simulink model "benchmark"  (Simulink 7.9)
settings: Solver=ode45  StartTime=0.0  StopTime=1000  RelTol=1e-3
undefined symbols (not defined in the file or in its folder): sigbuilder_block
libraries: libturbines x10, simulink x1
(part 2 of 3)

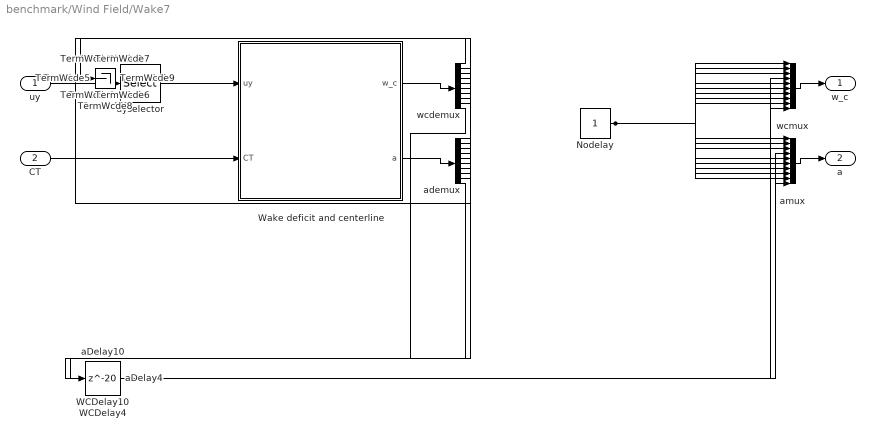
== system benchmark/Wind Field/Wake7 ==
  uy : Inport
  CT : Inport  Port=2
  Nodelay : Constant
  TermAde{1..3,5..9} (8 alike) : Terminator
  TermWcde{1..3,5..9} (8 alike) : Terminator
  WCDelay10 : Delay  DelayLength=20  InitialCondition=1500  InputProcessing=Inherited  SampleTime=1
  WCDelay4 : Delay  DelayLength=20  InitialCondition=1500  InputProcessing=Inherited  SampleTime=1
  Wake deficit and centerline : MATLAB Function  TreatAsAtomicUnit=on
  aDelay10 : Delay  DelayLength=20  InitialCondition=1  InputProcessing=Inherited  SampleTime=1
  aDelay4 : Delay  DelayLength=20  InitialCondition=1  InputProcessing=Inherited  SampleTime=1
  ademux : Demux  Outputs=10
  amux : Mux  Inputs=10
  uyselector : Selector  NumberOfDimensions=2  InputPortWidth=3  IndexOptions=Select all,Index vector (dialog)  Indices=[1 3],p.wt.num  OutputSizes=1,1
  wcdemux : Demux  Outputs=10
  wcmux : Mux  Inputs=10
  w_c : Outport
  a : Outport  Port=2
  wires:
  amux/1 -> a/1
  wcmux/1 -> w_c/1
  Wake deficit and centerline/2(a) -> ademux/1
  Wake deficit and centerline/1(w_c) -> wcdemux/1
  uyselector/1 -> Wake deficit and centerline/1(uy)
  uy/1 -> uyselector/1
  CT/1 -> Wake deficit and centerline/2(CT)
  ademux/1 -> TermAde1/1
  wcdemux/1 -> TermWcde1/1
  Nodelay/1 -> amux/1, wcmux/1, amux/2, wcmux/2, amux/3, wcmux/3, amux/5, wcmux/5, amux/6, wcmux/6, amux/7, wcmux/7, amux/8, wcmux/8, amux/9, wcmux/9
  ademux/2 -> TermAde2/1
  wcdemux/2 -> TermWcde2/1
  ademux/3 -> TermAde3/1
  wcdemux/3 -> TermWcde3/1
  ademux/4 -> aDelay4/1
  aDelay4/1 -> amux/4
  wcdemux/4 -> WCDelay4/1
  WCDelay4/1 -> wcmux/4
  ademux/5 -> TermAde5/1
  wcdemux/5 -> TermWcde5/1
  ademux/6 -> TermAde6/1
  wcdemux/6 -> TermWcde6/1
  ademux/7 -> TermAde7/1
  wcdemux/7 -> TermWcde7/1
  ademux/8 -> TermAde8/1
  wcdemux/8 -> TermWcde8/1
  ademux/9 -> TermAde9/1
  wcdemux/9 -> TermWcde9/1
  ademux/10 -> aDelay10/1
  aDelay10/1 -> amux/10
  wcdemux/10 -> WCDelay10/1
  WCDelay10/1 -> wcmux/10

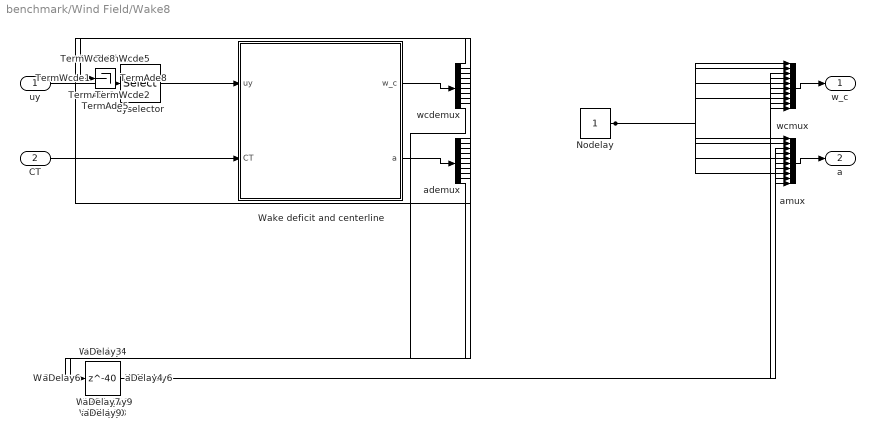
== system benchmark/Wind Field/Wake8 ==
  uy : Inport
  CT : Inport  Port=2
  Nodelay : Constant
  TermAde{1..2,5,8} (4 alike) : Terminator
  TermWcde{1..2,5,8} (4 alike) : Terminator
  WCDelay10 : Delay  DelayLength=80  InitialCondition=2000  InputProcessing=Inherited  SampleTime=1
  WCDelay3 : Delay  DelayLength=40  InitialCondition=2000  InputProcessing=Inherited  SampleTime=1
  WCDelay4 : Delay  DelayLength=80  InitialCondition=2000  InputProcessing=Inherited  SampleTime=1
  WCDelay6 : Delay  DelayLength=20  InitialCondition=2000  InputProcessing=Inherited  SampleTime=1
  WCDelay7 : Delay  DelayLength=60  InitialCondition=2000  InputProcessing=Inherited  SampleTime=1
  WCDelay9 : Delay  DelayLength=40  InitialCondition=2000  InputProcessing=Inherited  SampleTime=1
  Wake deficit and centerline : MATLAB Function  TreatAsAtomicUnit=on
  aDelay10 : Delay  DelayLength=80  InitialCondition=1  InputProcessing=Inherited  SampleTime=1
  aDelay3 : Delay  DelayLength=40  InitialCondition=1  InputProcessing=Inherited  SampleTime=1
  aDelay4 : Delay  DelayLength=80  InitialCondition=1  InputProcessing=Inherited  SampleTime=1
  aDelay6 : Delay  DelayLength=20  InitialCondition=1  InputProcessing=Inherited  SampleTime=1
  aDelay7 : Delay  DelayLength=60  InitialCondition=1  InputProcessing=Inherited  SampleTime=1
  aDelay9 : Delay  DelayLength=40  InitialCondition=1  InputProcessing=Inherited  SampleTime=1
  ademux : Demux  Outputs=10
  amux : Mux  Inputs=10
  uyselector : Selector  NumberOfDimensions=2  InputPortWidth=3  IndexOptions=Select all,Index vector (dialog)  Indices=[1 3],p.wt.num  OutputSizes=1,1
  wcdemux : Demux  Outputs=10
  wcmux : Mux  Inputs=10
  w_c : Outport
  a : Outport  Port=2
  wires:
  amux/1 -> a/1
  wcmux/1 -> w_c/1
  Wake deficit and centerline/2(a) -> ademux/1
  Wake deficit and centerline/1(w_c) -> wcdemux/1
  uyselector/1 -> Wake deficit and centerline/1(uy)
  uy/1 -> uyselector/1
  CT/1 -> Wake deficit and centerline/2(CT)
  ademux/1 -> TermAde1/1
  wcdemux/1 -> TermWcde1/1
  Nodelay/1 -> amux/1, wcmux/1, amux/2, wcmux/2, amux/5, wcmux/5, amux/8, wcmux/8
  ademux/2 -> TermAde2/1
  wcdemux/2 -> TermWcde2/1
  ademux/3 -> aDelay3/1
  aDelay3/1 -> amux/3
  wcdemux/3 -> WCDelay3/1
  WCDelay3/1 -> wcmux/3
  ademux/4 -> aDelay4/1
  aDelay4/1 -> amux/4
  wcdemux/4 -> WCDelay4/1
  WCDelay4/1 -> wcmux/4
  ademux/5 -> TermAde5/1
  wcdemux/5 -> TermWcde5/1
  ademux/6 -> aDelay6/1
  aDelay6/1 -> amux/6
  wcdemux/6 -> WCDelay6/1
  WCDelay6/1 -> wcmux/6
  ademux/7 -> aDelay7/1
  aDelay7/1 -> amux/7
  wcdemux/7 -> WCDelay7/1
  WCDelay7/1 -> wcmux/7
  ademux/8 -> TermAde8/1
  wcdemux/8 -> TermWcde8/1
  ademux/9 -> aDelay9/1
  aDelay9/1 -> amux/9
  wcdemux/9 -> WCDelay9/1
  WCDelay9/1 -> wcmux/9
  ademux/10 -> aDelay10/1
  aDelay10/1 -> amux/10
  wcdemux/10 -> WCDelay10/1
  WCDelay10/1 -> wcmux/10

== system benchmark/Wind Field/Wake9 ==
  uy : Inport
  CT : Inport  Port=2
  Nodelay : Constant
  TermAde{1..3,5..6,8..9} (7 alike) : Terminator
  TermWcde{1..3,5..6,8..9} (7 alike) : Terminator
  WCDelay10 : Delay  DelayLength=40  InitialCondition=2000  InputProcessing=Inherited  SampleTime=1
  WCDelay4 : Delay  DelayLength=40  InitialCondition=2000  InputProcessing=Inherited  SampleTime=1
  WCDelay7 : Delay  DelayLength=20  InitialCondition=2000  InputProcessing=Inherited  SampleTime=1
  Wake deficit and centerline : MATLAB Function  TreatAsAtomicUnit=on
  aDelay10 : Delay  DelayLength=40  InitialCondition=1  InputProcessing=Inherited  SampleTime=1
  aDelay4 : Delay  DelayLength=40  InitialCondition=1  InputProcessing=Inherited  SampleTime=1
  aDelay7 : Delay  DelayLength=20  InitialCondition=1  InputProcessing=Inherited  SampleTime=1
  ademux : Demux  Outputs=10
  amux : Mux  Inputs=10
  uyselector : Selector  NumberOfDimensions=2  InputPortWidth=3  IndexOptions=Select all,Index vector (dialog)  Indices=[1 3],p.wt.num  OutputSizes=1,1
  wcdemux : Demux  Outputs=10
  wcmux : Mux  Inputs=10
  w_c : Outport
  a : Outport  Port=2
  wires:
  amux/1 -> a/1
  wcmux/1 -> w_c/1
  Wake deficit and centerline/2(a) -> ademux/1
  Wake deficit and centerline/1(w_c) -> wcdemux/1
  uyselector/1 -> Wake deficit and centerline/1(uy)
  uy/1 -> uyselector/1
  CT/1 -> Wake deficit and centerline/2(CT)
  ademux/1 -> TermAde1/1
  wcdemux/1 -> TermWcde1/1
  Nodelay/1 -> amux/1, wcmux/1, amux/2, wcmux/2, amux/3, wcmux/3, amux/5, wcmux/5, amux/6, wcmux/6, amux/8, wcmux/8, amux/9, wcmux/9
  ademux/2 -> TermAde2/1
  wcdemux/2 -> TermWcde2/1
  ademux/3 -> TermAde3/1
  wcdemux/3 -> TermWcde3/1
  ademux/4 -> aDelay4/1
  aDelay4/1 -> amux/4
  wcdemux/4 -> WCDelay4/1
  WCDelay4/1 -> wcmux/4
  ademux/5 -> TermAde5/1
  wcdemux/5 -> TermWcde5/1
  ademux/6 -> TermAde6/1
  wcdemux/6 -> TermWcde6/1
  ademux/7 -> aDelay7/1
  aDelay7/1 -> amux/7
  wcdemux/7 -> WCDelay7/1
  WCDelay7/1 -> wcmux/7
  ademux/8 -> TermAde8/1
  wcdemux/8 -> TermWcde8/1
  ademux/9 -> TermAde9/1
  wcdemux/9 -> TermWcde9/1
  ademux/10 -> aDelay10/1
  aDelay10/1 -> amux/10
  wcdemux/10 -> WCDelay10/1
  WCDelay10/1 -> wcmux/10

== system benchmark/Wind Field/Wind Speed1 ==
  ux : Inport
  w_c : Inport  Port=2
  a : Inport  Port=3
  Signal Conversion : SignalConversion  ConversionOutput=Contiguous copy
  Signal Conversion1 : SignalConversion  ConversionOutput=Contiguous copy
  Wind speed : MATLAB Function  TreatAsAtomicUnit=on
  aselector : Selector  NumberOfDimensions=2  InputPortWidth=3  IndexOptions=Index vector (dialog),Select all  Indices=p.wt.num,p.wt.num  OutputSizes=1,1
  uxselector : Selector  NumberOfDimensions=2  InputPortWidth=3  IndexOptions=Select all,Index vector (dialog)  Indices=[1 3],p.wt.num  OutputSizes=1,1
  wcselector : Selector  NumberOfDimensions=2  InputPortWidth=3  IndexOptions=Index vector (dialog),Select all  Indices=p.wt.num,p.wt.num  OutputSizes=1,1
  v_rot : Outport
  v_nac : Outport  Port=2
  wires:
  Signal Conversion1/1 -> v_nac/1
  Signal Conversion/1 -> v_rot/1
  uxselector/1 -> Wind speed/1(ux)
  aselector/1 -> Wind speed/3(a)
  a/1 -> aselector/1
  wcselector/1 -> Wind speed/2(w_c)
  w_c/1 -> wcselector/1
  ux/1 -> uxselector/1
  Wind speed/1(uavg) -> Signal Conversion/1
  Wind speed/2(upoint) -> Signal Conversion1/1

== system benchmark/Wind Field/Wind Speed10 ==
  ux : Inport
  w_c : Inport  Port=2
  a : Inport  Port=3
  Signal Conversion : SignalConversion  ConversionOutput=Contiguous copy
  Signal Conversion1 : SignalConversion  ConversionOutput=Contiguous copy
  Wind speed : MATLAB Function  TreatAsAtomicUnit=on
  aselector : Selector  NumberOfDimensions=2  InputPortWidth=3  IndexOptions=Index vector (dialog),Select all  Indices=p.wt.num,p.wt.num  OutputSizes=1,1
  uxselector : Selector  NumberOfDimensions=2  InputPortWidth=3  IndexOptions=Select all,Index vector (dialog)  Indices=[1 3],p.wt.num  OutputSizes=1,1
  wcselector : Selector  NumberOfDimensions=2  InputPortWidth=3  IndexOptions=Index vector (dialog),Select all  Indices=p.wt.num,p.wt.num  OutputSizes=1,1
  v_rot : Outport
  v_nac : Outport  Port=2
  wires:
  Signal Conversion1/1 -> v_nac/1
  Signal Conversion/1 -> v_rot/1
  uxselector/1 -> Wind speed/1(ux)
  aselector/1 -> Wind speed/3(a)
  a/1 -> aselector/1
  wcselector/1 -> Wind speed/2(w_c)
  w_c/1 -> wcselector/1
  ux/1 -> uxselector/1
  Wind speed/1(uavg) -> Signal Conversion/1
  Wind speed/2(upoint) -> Signal Conversion1/1

== system benchmark/Wind Field/Wind Speed2 ==
  ux : Inport
  w_c : Inport  Port=2
  a : Inport  Port=3
  Signal Conversion : SignalConversion  ConversionOutput=Contiguous copy
  Signal Conversion1 : SignalConversion  ConversionOutput=Contiguous copy
  Wind speed : MATLAB Function  TreatAsAtomicUnit=on
  aselector : Selector  NumberOfDimensions=2  InputPortWidth=3  IndexOptions=Index vector (dialog),Select all  Indices=p.wt.num,p.wt.num  OutputSizes=1,1
  uxselector : Selector  NumberOfDimensions=2  InputPortWidth=3  IndexOptions=Select all,Index vector (dialog)  Indices=[1 3],p.wt.num  OutputSizes=1,1
  wcselector : Selector  NumberOfDimensions=2  InputPortWidth=3  IndexOptions=Index vector (dialog),Select all  Indices=p.wt.num,p.wt.num  OutputSizes=1,1
  v_rot : Outport
  v_nac : Outport  Port=2
  wires:
  Signal Conversion1/1 -> v_nac/1
  Signal Conversion/1 -> v_rot/1
  uxselector/1 -> Wind speed/1(ux)
  aselector/1 -> Wind speed/3(a)
  a/1 -> aselector/1
  wcselector/1 -> Wind speed/2(w_c)
  w_c/1 -> wcselector/1
  ux/1 -> uxselector/1
  Wind speed/1(uavg) -> Signal Conversion/1
  Wind speed/2(upoint) -> Signal Conversion1/1

== system benchmark/Wind Field/Wind Speed3 ==
  ux : Inport
  w_c : Inport  Port=2
  a : Inport  Port=3
  Signal Conversion : SignalConversion  ConversionOutput=Contiguous copy
  Signal Conversion1 : SignalConversion  ConversionOutput=Contiguous copy
  Wind speed : MATLAB Function  TreatAsAtomicUnit=on
  aselector : Selector  NumberOfDimensions=2  InputPortWidth=3  IndexOptions=Index vector (dialog),Select all  Indices=p.wt.num,p.wt.num  OutputSizes=1,1
  uxselector : Selector  NumberOfDimensions=2  InputPortWidth=3  IndexOptions=Select all,Index vector (dialog)  Indices=[1 3],p.wt.num  OutputSizes=1,1
  wcselector : Selector  NumberOfDimensions=2  InputPortWidth=3  IndexOptions=Index vector (dialog),Select all  Indices=p.wt.num,p.wt.num  OutputSizes=1,1
  v_rot : Outport
  v_nac : Outport  Port=2
  wires:
  Signal Conversion1/1 -> v_nac/1
  Signal Conversion/1 -> v_rot/1
  uxselector/1 -> Wind speed/1(ux)
  aselector/1 -> Wind speed/3(a)
  a/1 -> aselector/1
  wcselector/1 -> Wind speed/2(w_c)
  w_c/1 -> wcselector/1
  ux/1 -> uxselector/1
  Wind speed/1(uavg) -> Signal Conversion/1
  Wind speed/2(upoint) -> Signal Conversion1/1

== system benchmark/Wind Field/Wind Speed4 ==
  ux : Inport
  w_c : Inport  Port=2
  a : Inport  Port=3
  Signal Conversion : SignalConversion  ConversionOutput=Contiguous copy
  Signal Conversion1 : SignalConversion  ConversionOutput=Contiguous copy
  Wind speed : MATLAB Function  TreatAsAtomicUnit=on
  aselector : Selector  NumberOfDimensions=2  InputPortWidth=3  IndexOptions=Index vector (dialog),Select all  Indices=p.wt.num,p.wt.num  OutputSizes=1,1
  uxselector : Selector  NumberOfDimensions=2  InputPortWidth=3  IndexOptions=Select all,Index vector (dialog)  Indices=[1 3],p.wt.num  OutputSizes=1,1
  wcselector : Selector  NumberOfDimensions=2  InputPortWidth=3  IndexOptions=Index vector (dialog),Select all  Indices=p.wt.num,p.wt.num  OutputSizes=1,1
  v_rot : Outport
  v_nac : Outport  Port=2
  wires:
  Signal Conversion1/1 -> v_nac/1
  Signal Conversion/1 -> v_rot/1
  uxselector/1 -> Wind speed/1(ux)
  aselector/1 -> Wind speed/3(a)
  a/1 -> aselector/1
  wcselector/1 -> Wind speed/2(w_c)
  w_c/1 -> wcselector/1
  ux/1 -> uxselector/1
  Wind speed/1(uavg) -> Signal Conversion/1
  Wind speed/2(upoint) -> Signal Conversion1/1

== system benchmark/Wind Field/Wind Speed5 ==
  ux : Inport
  w_c : Inport  Port=2
  a : Inport  Port=3
  Signal Conversion : SignalConversion  ConversionOutput=Contiguous copy
  Signal Conversion1 : SignalConversion  ConversionOutput=Contiguous copy
  Wind speed : MATLAB Function  TreatAsAtomicUnit=on
  aselector : Selector  NumberOfDimensions=2  InputPortWidth=3  IndexOptions=Index vector (dialog),Select all  Indices=p.wt.num,p.wt.num  OutputSizes=1,1
  uxselector : Selector  NumberOfDimensions=2  InputPortWidth=3  IndexOptions=Select all,Index vector (dialog)  Indices=[1 3],p.wt.num  OutputSizes=1,1
  wcselector : Selector  NumberOfDimensions=2  InputPortWidth=3  IndexOptions=Index vector (dialog),Select all  Indices=p.wt.num,p.wt.num  OutputSizes=1,1
  v_rot : Outport
  v_nac : Outport  Port=2
  wires:
  Signal Conversion1/1 -> v_nac/1
  Signal Conversion/1 -> v_rot/1
  uxselector/1 -> Wind speed/1(ux)
  aselector/1 -> Wind speed/3(a)
  a/1 -> aselector/1
  wcselector/1 -> Wind speed/2(w_c)
  w_c/1 -> wcselector/1
  ux/1 -> uxselector/1
  Wind speed/1(uavg) -> Signal Conversion/1
  Wind speed/2(upoint) -> Signal Conversion1/1

== system benchmark/Wind Field/Wind Speed6 ==
  ux : Inport
  w_c : Inport  Port=2
  a : Inport  Port=3
  Signal Conversion : SignalConversion  ConversionOutput=Contiguous copy
  Signal Conversion1 : SignalConversion  ConversionOutput=Contiguous copy
  Wind speed : MATLAB Function  TreatAsAtomicUnit=on
  aselector : Selector  NumberOfDimensions=2  InputPortWidth=3  IndexOptions=Index vector (dialog),Select all  Indices=p.wt.num,p.wt.num  OutputSizes=1,1
  uxselector : Selector  NumberOfDimensions=2  InputPortWidth=3  IndexOptions=Select all,Index vector (dialog)  Indices=[1 3],p.wt.num  OutputSizes=1,1
  wcselector : Selector  NumberOfDimensions=2  InputPortWidth=3  IndexOptions=Index vector (dialog),Select all  Indices=p.wt.num,p.wt.num  OutputSizes=1,1
  v_rot : Outport
  v_nac : Outport  Port=2
  wires:
  Signal Conversion1/1 -> v_nac/1
  Signal Conversion/1 -> v_rot/1
  uxselector/1 -> Wind speed/1(ux)
  aselector/1 -> Wind speed/3(a)
  a/1 -> aselector/1
  wcselector/1 -> Wind speed/2(w_c)
  w_c/1 -> wcselector/1
  ux/1 -> uxselector/1
  Wind speed/1(uavg) -> Signal Conversion/1
  Wind speed/2(upoint) -> Signal Conversion1/1

== system benchmark/Wind Field/Wind Speed7 ==
  ux : Inport
  w_c : Inport  Port=2
  a : Inport  Port=3
  Signal Conversion : SignalConversion  ConversionOutput=Contiguous copy
  Signal Conversion1 : SignalConversion  ConversionOutput=Contiguous copy
  Wind speed : MATLAB Function  TreatAsAtomicUnit=on
  aselector : Selector  NumberOfDimensions=2  InputPortWidth=3  IndexOptions=Index vector (dialog),Select all  Indices=p.wt.num,p.wt.num  OutputSizes=1,1
  uxselector : Selector  NumberOfDimensions=2  InputPortWidth=3  IndexOptions=Select all,Index vector (dialog)  Indices=[1 3],p.wt.num  OutputSizes=1,1
  wcselector : Selector  NumberOfDimensions=2  InputPortWidth=3  IndexOptions=Index vector (dialog),Select all  Indices=p.wt.num,p.wt.num  OutputSizes=1,1
  v_rot : Outport
  v_nac : Outport  Port=2
  wires:
  Signal Conversion1/1 -> v_nac/1
  Signal Conversion/1 -> v_rot/1
  uxselector/1 -> Wind speed/1(ux)
  aselector/1 -> Wind speed/3(a)
  a/1 -> aselector/1
  wcselector/1 -> Wind speed/2(w_c)
  w_c/1 -> wcselector/1
  ux/1 -> uxselector/1
  Wind speed/1(uavg) -> Signal Conversion/1
  Wind speed/2(upoint) -> Signal Conversion1/1

== system benchmark/Wind Field/Wind Speed8 ==
  ux : Inport
  w_c : Inport  Port=2
  a : Inport  Port=3
  Signal Conversion : SignalConversion  ConversionOutput=Contiguous copy
  Signal Conversion1 : SignalConversion  ConversionOutput=Contiguous copy
  Wind speed : MATLAB Function  TreatAsAtomicUnit=on
  aselector : Selector  NumberOfDimensions=2  InputPortWidth=3  IndexOptions=Index vector (dialog),Select all  Indices=p.wt.num,p.wt.num  OutputSizes=1,1
  uxselector : Selector  NumberOfDimensions=2  InputPortWidth=3  IndexOptions=Select all,Index vector (dialog)  Indices=[1 3],p.wt.num  OutputSizes=1,1
  wcselector : Selector  NumberOfDimensions=2  InputPortWidth=3  IndexOptions=Index vector (dialog),Select all  Indices=p.wt.num,p.wt.num  OutputSizes=1,1
  v_rot : Outport
  v_nac : Outport  Port=2
  wires:
  Signal Conversion1/1 -> v_nac/1
  Signal Conversion/1 -> v_rot/1
  uxselector/1 -> Wind speed/1(ux)
  aselector/1 -> Wind speed/3(a)
  a/1 -> aselector/1
  wcselector/1 -> Wind speed/2(w_c)
  w_c/1 -> wcselector/1
  ux/1 -> uxselector/1
  Wind speed/1(uavg) -> Signal Conversion/1
  Wind speed/2(upoint) -> Signal Conversion1/1

== system benchmark/Wind Field/Wind Speed9 ==
  ux : Inport
  w_c : Inport  Port=2
  a : Inport  Port=3
  Signal Conversion : SignalConversion  ConversionOutput=Contiguous copy
  Signal Conversion1 : SignalConversion  ConversionOutput=Contiguous copy
  Wind speed : MATLAB Function  TreatAsAtomicUnit=on
  aselector : Selector  NumberOfDimensions=2  InputPortWidth=3  IndexOptions=Index vector (dialog),Select all  Indices=p.wt.num,p.wt.num  OutputSizes=1,1
  uxselector : Selector  NumberOfDimensions=2  InputPortWidth=3  IndexOptions=Select all,Index vector (dialog)  Indices=[1 3],p.wt.num  OutputSizes=1,1
  wcselector : Selector  NumberOfDimensions=2  InputPortWidth=3  IndexOptions=Index vector (dialog),Select all  Indices=p.wt.num,p.wt.num  OutputSizes=1,1
  v_rot : Outport
  v_nac : Outport  Port=2
  wires:
  Signal Conversion1/1 -> v_nac/1
  Signal Conversion/1 -> v_rot/1
  uxselector/1 -> Wind speed/1(ux)
  aselector/1 -> Wind speed/3(a)
  a/1 -> aselector/1
  wcselector/1 -> Wind speed/2(w_c)
  w_c/1 -> wcselector/1
  ux/1 -> uxselector/1
  Wind speed/1(uavg) -> Signal Conversion/1
  Wind speed/2(upoint) -> Signal Conversion1/1

== matlab function Wind Field/Wake2/Wake deficit and centerline ==
  data: uy input double; CT input double; p parameter double; w_c output double; a output double
function [w_c,a]=wake(uy,CT,p)
    % Todo: Change length(uy)*grid_size to farm size?
    %       Change such that parm is passed directly

    w_c=zeros(length(p.farm.x),1);
    a=ones(length(p.farm.x),1);
    
    %Find wake center and deficit for all other turbines
    for i=1:length(p.farm.x)
        if(p.farm.x(i)-p.wt.x>0) %Is turbine P downwind?
            w_c(i)=max(min(wakecenter(uy,p.farm.x(i),p),p.grid.ysize),1);
            a(i)=deficit(CT,p.farm.x(i),p);
        else
            w_c(i)=p.wt.y;
            a(i)=1;
        end
    end
end


function wc=wakecenter(uy,px,p)
    
    %computer number of grid points to px
    it=ceil((px-p.wt.x)/p.grid.size);
    
    wc=p.wt.y; %wake center starts at turbine position
    
    for i=1:it %Compute the meandering for each grid point
        uysum=0;
        %compute the grid number for low meandering edge
        m_min=max(floor((wc-p.wt.meand.r(i))/p.grid.size)+1,1); 
        %compute the grid number for the high meandering edge
        m_max=min(ceil((wc+p.wt.meand.r(i))/p.grid.size)+1,length(uy)); 
        
        %average transversal velocity over the wake area
        for j=m_min:m_max
            uysum=uysum+uy(j);
        end
        uyavg=uysum/(m_max-m_min+1);
        
        % wake center filtered
        % Should be calculated from mean air speed vs uyavg and lowpass filtered
        % Redo filter computation
        % yeff=ts*uyavg=grid.size/uxmean*uyavg
        % wc=wc+alpha*uyavg
        
        %Uncomment to reproduce bug
        %wc=p.wt.y;
        
        %Uncomment to reproduce meandering limits
        %wc=min(max(wc,p.wt.y-150),p.wt.y+150);
        
        %Notice here ts such that wake moves with uyavg speed
        wc=wc+p.ts*uyavg;
    end
end

function a=deficit(CT,px,p)
    %wake deficit at px-wtx distance from turbine
    a=1-.5*CT/(1+(px-p.wt.x)/(4*p.wt.rotor.r));
end

== matlab function Wind Field/Wind Speed2/Wind speed ==
  data: ux input double; w_c input double; a input double; p parameter double; uavg output double; upoint output double
function [uavg,upoint]=windspeed(ux,w_c,a,p)
% ux    is the wind vector at the x position of the turbine
% w_c   is the vector of wake centers from the other turbins at the x position of the turbine
% a     is the vector of velocity deficits at this turbine from the other turbines
% P     is a structure containing parameters  
   
%Initialise variables
uxsum=0;
av=ones(p.grid.ysize,1);

%Merge the wake from all turbines upwind
for i=1:length(p.farm.x)
    if(p.farm.x(i)<p.wt.x) %only compute deficit effect from upstream turbines

        %compute the grid number for low meandering edge
        mn=floor((w_c(i)-p.wt.exprad(i))/p.grid.size)+1;
        m_min=max(mn,1); 
        %compute the grid number for the high meandering edge
        mx=floor((w_c(i)+p.wt.exprad(i))/p.grid.size)+1;
        m_max=min(mx,floor(p.grid.ysize/p.grid.size)+1);

        %Merge wakes
        for j=m_min:m_max
            av(j)=av(j)*a(i);
        end
    end
end
    


%Rotor grid points - Todo move to farm setup
%compute the grid number for low rotor edge
r_min=floor((p.wt.y-p.wt.rotor.r)/p.grid.size)+1; 
%compute the grid number for the high rotor edge
r_max=floor((p.wt.y+p.wt.rotor.r)/p.grid.size)+1;

%Sum the wake corrected x-velocities over the rotor dish area
for i=r_min:r_max
    uxsum=uxsum+ux(i)*av(i);
end

%Compute the average
uavg=uxsum/(r_max-r_min+1);

%Extract the wake corrected velocity at the nacelle
upoint=ux(floor(p.wt.y/p.grid.size)+1)*av(floor(p.wt.y/p.grid.size)+1);
    
end

== matlab function Wind Field/Wake1/Wake deficit and centerline ==
  data: uy input double; CT input double; p parameter double; w_c output double; a output double
function [w_c,a]=wake(uy,CT,p)
    % Todo: Change length(uy)*grid_size to farm size?
    %       Change such that parm is passed directly

    w_c=zeros(length(p.farm.x),1);
    a=ones(length(p.farm.x),1);
    
    %Find wake center and deficit for all other turbines
    for i=1:length(p.farm.x)
        if(p.farm.x(i)-p.wt.x>0) %Is turbine P downwind?
            w_c(i)=max(min(wakecenter(uy,p.farm.x(i),p),p.grid.ysize),1);
            a(i)=deficit(CT,p.farm.x(i),p);
        else
            w_c(i)=p.wt.y;
            a(i)=1;
        end
    end
end


function wc=wakecenter(uy,px,p)
    
    %computer number of grid points to px
    it=ceil((px-p.wt.x)/p.grid.size);
    
    wc=p.wt.y; %wake center starts at turbine position
    
    for i=1:it %Compute the meandering for each grid point
        uysum=0;
        %compute the grid number for low meandering edge
        m_min=max(floor((wc-p.wt.meand.r(i))/p.grid.size)+1,1); 
        %compute the grid number for the high meandering edge
        m_max=min(ceil((wc+p.wt.meand.r(i))/p.grid.size)+1,length(uy)); 
        
        %average transversal velocity over the wake area
        for j=m_min:m_max
            uysum=uysum+uy(j);
        end
        uyavg=uysum/(m_max-m_min+1);
        
        % wake center filtered
        % Should be calculated from mean air speed vs uyavg and lowpass filtered
        % Redo filter computation
        % yeff=ts*uyavg=grid.size/uxmean*uyavg
        % wc=wc+alpha*uyavg
        
        %Uncomment to reproduce bug
        %wc=p.wt.y;
        
        %Uncomment to reproduce meandering limits
        %wc=min(max(wc,p.wt.y-150),p.wt.y+150);
        
        %Notice here ts such that wake moves with uyavg speed
        wc=wc+p.ts*uyavg;
    end
end

function a=deficit(CT,px,p)
    %wake deficit at px-wtx distance from turbine
    a=1-.5*CT/(1+(px-p.wt.x)/(4*p.wt.rotor.r));
end

== matlab function Wind Field/Wind Speed1/Wind speed ==
  data: ux input double; w_c input double; a input double; p parameter double; uavg output double; upoint output double
function [uavg,upoint]=windspeed(ux,w_c,a,p)
% ux    is the wind vector at the x position of the turbine
% w_c   is the vector of wake centers from the other turbins at the x position of the turbine
% a     is the vector of velocity deficits at this turbine from the other turbines
% P     is a structure containing parameters  
   
%Initialise variables
uxsum=0;
av=ones(p.grid.ysize,1);

%Merge the wake from all turbines upwind
for i=1:length(p.farm.x)
    if(p.farm.x(i)<p.wt.x) %only compute deficit effect from upstream turbines

        %compute the grid number for low meandering edge
        mn=floor((w_c(i)-p.wt.exprad(i))/p.grid.size)+1;
        m_min=max(mn,1); 
        %compute the grid number for the high meandering edge
        mx=floor((w_c(i)+p.wt.exprad(i))/p.grid.size)+1;
        m_max=min(mx,floor(p.grid.ysize/p.grid.size)+1);

        %Merge wakes
        for j=m_min:m_max
            av(j)=av(j)*a(i);
        end
    end
end
    


%Rotor grid points - Todo move to farm setup
%compute the grid number for low rotor edge
r_min=floor((p.wt.y-p.wt.rotor.r)/p.grid.size)+1; 
%compute the grid number for the high rotor edge
r_max=floor((p.wt.y+p.wt.rotor.r)/p.grid.size)+1;

%Sum the wake corrected x-velocities over the rotor dish area
for i=r_min:r_max
    uxsum=uxsum+ux(i)*av(i);
end

%Compute the average
uavg=uxsum/(r_max-r_min+1);

%Extract the wake corrected velocity at the nacelle
upoint=ux(floor(p.wt.y/p.grid.size)+1)*av(floor(p.wt.y/p.grid.size)+1);
    
end

== matlab function Wind Field/Wake3/Wake deficit and centerline ==
  data: uy input double; CT input double; p parameter double; w_c output double; a output double
function [w_c,a]=wake(uy,CT,p)
    % Todo: Change length(uy)*grid_size to farm size?
    %       Change such that parm is passed directly

    w_c=zeros(length(p.farm.x),1);
    a=ones(length(p.farm.x),1);
    
    %Find wake center and deficit for all other turbines
    for i=1:length(p.farm.x)
        if(p.farm.x(i)-p.wt.x>0) %Is turbine P downwind?
            w_c(i)=max(min(wakecenter(uy,p.farm.x(i),p),p.grid.ysize),1);
            a(i)=deficit(CT,p.farm.x(i),p);
        else
            w_c(i)=p.wt.y;
            a(i)=1;
        end
    end
end


function wc=wakecenter(uy,px,p)
    
    %computer number of grid points to px
    it=ceil((px-p.wt.x)/p.grid.size);
    
    wc=p.wt.y; %wake center starts at turbine position
    
    for i=1:it %Compute the meandering for each grid point
        uysum=0;
        %compute the grid number for low meandering edge
        m_min=max(floor((wc-p.wt.meand.r(i))/p.grid.size)+1,1); 
        %compute the grid number for the high meandering edge
        m_max=min(ceil((wc+p.wt.meand.r(i))/p.grid.size)+1,length(uy)); 
        
        %average transversal velocity over the wake area
        for j=m_min:m_max
            uysum=uysum+uy(j);
        end
        uyavg=uysum/(m_max-m_min+1);
        
        % wake center filtered
        % Should be calculated from mean air speed vs uyavg and lowpass filtered
        % Redo filter computation
        % yeff=ts*uyavg=grid.size/uxmean*uyavg
        % wc=wc+alpha*uyavg
        
        %Uncomment to reproduce bug
        %wc=p.wt.y;
        
        %Uncomment to reproduce meandering limits
        %wc=min(max(wc,p.wt.y-150),p.wt.y+150);
        
        %Notice here ts such that wake moves with uyavg speed
        wc=wc+p.ts*uyavg;
    end
end

function a=deficit(CT,px,p)
    %wake deficit at px-wtx distance from turbine
    a=1-.5*CT/(1+(px-p.wt.x)/(4*p.wt.rotor.r));
end

== matlab function Wind Field/Wind Speed3/Wind speed ==
  data: ux input double; w_c input double; a input double; p parameter double; uavg output double; upoint output double
function [uavg,upoint]=windspeed(ux,w_c,a,p)
% ux    is the wind vector at the x position of the turbine
% w_c   is the vector of wake centers from the other turbins at the x position of the turbine
% a     is the vector of velocity deficits at this turbine from the other turbines
% P     is a structure containing parameters  
   
%Initialise variables
uxsum=0;
av=ones(p.grid.ysize,1);

%Merge the wake from all turbines upwind
for i=1:length(p.farm.x)
    if(p.farm.x(i)<p.wt.x) %only compute deficit effect from upstream turbines

        %compute the grid number for low meandering edge
        mn=floor((w_c(i)-p.wt.exprad(i))/p.grid.size)+1;
        m_min=max(mn,1); 
        %compute the grid number for the high meandering edge
        mx=floor((w_c(i)+p.wt.exprad(i))/p.grid.size)+1;
        m_max=min(mx,floor(p.grid.ysize/p.grid.size)+1);

        %Merge wakes
        for j=m_min:m_max
            av(j)=av(j)*a(i);
        end
    end
end
    


%Rotor grid points - Todo move to farm setup
%compute the grid number for low rotor edge
r_min=floor((p.wt.y-p.wt.rotor.r)/p.grid.size)+1; 
%compute the grid number for the high rotor edge
r_max=floor((p.wt.y+p.wt.rotor.r)/p.grid.size)+1;

%Sum the wake corrected x-velocities over the rotor dish area
for i=r_min:r_max
    uxsum=uxsum+ux(i)*av(i);
end

%Compute the average
uavg=uxsum/(r_max-r_min+1);

%Extract the wake corrected velocity at the nacelle
upoint=ux(floor(p.wt.y/p.grid.size)+1)*av(floor(p.wt.y/p.grid.size)+1);
    
end

== matlab function Wind Field/Wake4/Wake deficit and centerline ==
  data: uy input double; CT input double; p parameter double; w_c output double; a output double
function [w_c,a]=wake(uy,CT,p)
    % Todo: Change length(uy)*grid_size to farm size?
    %       Change such that parm is passed directly

    w_c=zeros(length(p.farm.x),1);
    a=ones(length(p.farm.x),1);
    
    %Find wake center and deficit for all other turbines
    for i=1:length(p.farm.x)
        if(p.farm.x(i)-p.wt.x>0) %Is turbine P downwind?
            w_c(i)=max(min(wakecenter(uy,p.farm.x(i),p),p.grid.ysize),1);
            a(i)=deficit(CT,p.farm.x(i),p);
        else
            w_c(i)=p.wt.y;
            a(i)=1;
        end
    end
end


function wc=wakecenter(uy,px,p)
    
    %computer number of grid points to px
    it=ceil((px-p.wt.x)/p.grid.size);
    
    wc=p.wt.y; %wake center starts at turbine position
    
    for i=1:it %Compute the meandering for each grid point
        uysum=0;
        %compute the grid number for low meandering edge
        m_min=max(floor((wc-p.wt.meand.r(i))/p.grid.size)+1,1); 
        %compute the grid number for the high meandering edge
        m_max=min(ceil((wc+p.wt.meand.r(i))/p.grid.size)+1,length(uy)); 
        
        %average transversal velocity over the wake area
        for j=m_min:m_max
            uysum=uysum+uy(j);
        end
        uyavg=uysum/(m_max-m_min+1);
        
        % wake center filtered
        % Should be calculated from mean air speed vs uyavg and lowpass filtered
        % Redo filter computation
        % yeff=ts*uyavg=grid.size/uxmean*uyavg
        % wc=wc+alpha*uyavg
        
        %Uncomment to reproduce bug
        %wc=p.wt.y;
        
        %Uncomment to reproduce meandering limits
        %wc=min(max(wc,p.wt.y-150),p.wt.y+150);
        
        %Notice here ts such that wake moves with uyavg speed
        wc=wc+p.ts*uyavg;
    end
end

function a=deficit(CT,px,p)
    %wake deficit at px-wtx distance from turbine
    a=1-.5*CT/(1+(px-p.wt.x)/(4*p.wt.rotor.r));
end

== matlab function Wind Field/Wind Speed4/Wind speed ==
  data: ux input double; w_c input double; a input double; p parameter double; uavg output double; upoint output double
function [uavg,upoint]=windspeed(ux,w_c,a,p)
% ux    is the wind vector at the x position of the turbine
% w_c   is the vector of wake centers from the other turbins at the x position of the turbine
% a     is the vector of velocity deficits at this turbine from the other turbines
% P     is a structure containing parameters  
   
%Initialise variables
uxsum=0;
av=ones(p.grid.ysize,1);

%Merge the wake from all turbines upwind
for i=1:length(p.farm.x)
    if(p.farm.x(i)<p.wt.x) %only compute deficit effect from upstream turbines

        %compute the grid number for low meandering edge
        mn=floor((w_c(i)-p.wt.exprad(i))/p.grid.size)+1;
        m_min=max(mn,1); 
        %compute the grid number for the high meandering edge
        mx=floor((w_c(i)+p.wt.exprad(i))/p.grid.size)+1;
        m_max=min(mx,floor(p.grid.ysize/p.grid.size)+1);

        %Merge wakes
        for j=m_min:m_max
            av(j)=av(j)*a(i);
        end
    end
end
    


%Rotor grid points - Todo move to farm setup
%compute the grid number for low rotor edge
r_min=floor((p.wt.y-p.wt.rotor.r)/p.grid.size)+1; 
%compute the grid number for the high rotor edge
r_max=floor((p.wt.y+p.wt.rotor.r)/p.grid.size)+1;

%Sum the wake corrected x-velocities over the rotor dish area
for i=r_min:r_max
    uxsum=uxsum+ux(i)*av(i);
end

%Compute the average
uavg=uxsum/(r_max-r_min+1);

%Extract the wake corrected velocity at the nacelle
upoint=ux(floor(p.wt.y/p.grid.size)+1)*av(floor(p.wt.y/p.grid.size)+1);
    
end

== matlab function Wind Field/Wake5/Wake deficit and centerline ==
  data: uy input double; CT input double; p parameter double; w_c output double; a output double
function [w_c,a]=wake(uy,CT,p)
    % Todo: Change length(uy)*grid_size to farm size?
    %       Change such that parm is passed directly

    w_c=zeros(length(p.farm.x),1);
    a=ones(length(p.farm.x),1);
    
    %Find wake center and deficit for all other turbines
    for i=1:length(p.farm.x)
        if(p.farm.x(i)-p.wt.x>0) %Is turbine P downwind?
            w_c(i)=max(min(wakecenter(uy,p.farm.x(i),p),p.grid.ysize),1);
            a(i)=deficit(CT,p.farm.x(i),p);
        else
            w_c(i)=p.wt.y;
            a(i)=1;
        end
    end
end


function wc=wakecenter(uy,px,p)
    
    %computer number of grid points to px
    it=ceil((px-p.wt.x)/p.grid.size);
    
    wc=p.wt.y; %wake center starts at turbine position
    
    for i=1:it %Compute the meandering for each grid point
        uysum=0;
        %compute the grid number for low meandering edge
        m_min=max(floor((wc-p.wt.meand.r(i))/p.grid.size)+1,1); 
        %compute the grid number for the high meandering edge
        m_max=min(ceil((wc+p.wt.meand.r(i))/p.grid.size)+1,length(uy)); 
        
        %average transversal velocity over the wake area
        for j=m_min:m_max
            uysum=uysum+uy(j);
        end
        uyavg=uysum/(m_max-m_min+1);
        
        % wake center filtered
        % Should be calculated from mean air speed vs uyavg and lowpass filtered
        % Redo filter computation
        % yeff=ts*uyavg=grid.size/uxmean*uyavg
        % wc=wc+alpha*uyavg
        
        %Uncomment to reproduce bug
        %wc=p.wt.y;
        
        %Uncomment to reproduce meandering limits
        %wc=min(max(wc,p.wt.y-150),p.wt.y+150);
        
        %Notice here ts such that wake moves with uyavg speed
        wc=wc+p.ts*uyavg;
    end
end

function a=deficit(CT,px,p)
    %wake deficit at px-wtx distance from turbine
    a=1-.5*CT/(1+(px-p.wt.x)/(4*p.wt.rotor.r));
end

== matlab function Wind Field/Wind Speed5/Wind speed ==
  data: ux input double; w_c input double; a input double; p parameter double; uavg output double; upoint output double
function [uavg,upoint]=windspeed(ux,w_c,a,p)
% ux    is the wind vector at the x position of the turbine
% w_c   is the vector of wake centers from the other turbins at the x position of the turbine
% a     is the vector of velocity deficits at this turbine from the other turbines
% P     is a structure containing parameters  
   
%Initialise variables
uxsum=0;
av=ones(p.grid.ysize,1);

%Merge the wake from all turbines upwind
for i=1:length(p.farm.x)
    if(p.farm.x(i)<p.wt.x) %only compute deficit effect from upstream turbines

        %compute the grid number for low meandering edge
        mn=floor((w_c(i)-p.wt.exprad(i))/p.grid.size)+1;
        m_min=max(mn,1); 
        %compute the grid number for the high meandering edge
        mx=floor((w_c(i)+p.wt.exprad(i))/p.grid.size)+1;
        m_max=min(mx,floor(p.grid.ysize/p.grid.size)+1);

        %Merge wakes
        for j=m_min:m_max
            av(j)=av(j)*a(i);
        end
    end
end
    


%Rotor grid points - Todo move to farm setup
%compute the grid number for low rotor edge
r_min=floor((p.wt.y-p.wt.rotor.r)/p.grid.size)+1; 
%compute the grid number for the high rotor edge
r_max=floor((p.wt.y+p.wt.rotor.r)/p.grid.size)+1;

%Sum the wake corrected x-velocities over the rotor dish area
for i=r_min:r_max
    uxsum=uxsum+ux(i)*av(i);
end

%Compute the average
uavg=uxsum/(r_max-r_min+1);

%Extract the wake corrected velocity at the nacelle
upoint=ux(floor(p.wt.y/p.grid.size)+1)*av(floor(p.wt.y/p.grid.size)+1);
    
end

== matlab function Wind Field/Wake6/Wake deficit and centerline ==
  data: uy input double; CT input double; p parameter double; w_c output double; a output double
function [w_c,a]=wake(uy,CT,p)
    % Todo: Change length(uy)*grid_size to farm size?
    %       Change such that parm is passed directly

    w_c=zeros(length(p.farm.x),1);
    a=ones(length(p.farm.x),1);
    
    %Find wake center and deficit for all other turbines
    for i=1:length(p.farm.x)
        if(p.farm.x(i)-p.wt.x>0) %Is turbine P downwind?
            w_c(i)=max(min(wakecenter(uy,p.farm.x(i),p),p.grid.ysize),1);
            a(i)=deficit(CT,p.farm.x(i),p);
        else
            w_c(i)=p.wt.y;
            a(i)=1;
        end
    end
end


function wc=wakecenter(uy,px,p)
    
    %computer number of grid points to px
    it=ceil((px-p.wt.x)/p.grid.size);
    
    wc=p.wt.y; %wake center starts at turbine position
    
    for i=1:it %Compute the meandering for each grid point
        uysum=0;
        %compute the grid number for low meandering edge
        m_min=max(floor((wc-p.wt.meand.r(i))/p.grid.size)+1,1); 
        %compute the grid number for the high meandering edge
        m_max=min(ceil((wc+p.wt.meand.r(i))/p.grid.size)+1,length(uy)); 
        
        %average transversal velocity over the wake area
        for j=m_min:m_max
            uysum=uysum+uy(j);
        end
        uyavg=uysum/(m_max-m_min+1);
        
        % wake center filtered
        % Should be calculated from mean air speed vs uyavg and lowpass filtered
        % Redo filter computation
        % yeff=ts*uyavg=grid.size/uxmean*uyavg
        % wc=wc+alpha*uyavg
        
        %Uncomment to reproduce bug
        %wc=p.wt.y;
        
        %Uncomment to reproduce meandering limits
        %wc=min(max(wc,p.wt.y-150),p.wt.y+150);
        
        %Notice here ts such that wake moves with uyavg speed
        wc=wc+p.ts*uyavg;
    end
end

function a=deficit(CT,px,p)
    %wake deficit at px-wtx distance from turbine
    a=1-.5*CT/(1+(px-p.wt.x)/(4*p.wt.rotor.r));
end

== matlab function Wind Field/Wind Speed6/Wind speed ==
  data: ux input double; w_c input double; a input double; p parameter double; uavg output double; upoint output double
function [uavg,upoint]=windspeed(ux,w_c,a,p)
% ux    is the wind vector at the x position of the turbine
% w_c   is the vector of wake centers from the other turbins at the x position of the turbine
% a     is the vector of velocity deficits at this turbine from the other turbines
% P     is a structure containing parameters  
   
%Initialise variables
uxsum=0;
av=ones(p.grid.ysize,1);

%Merge the wake from all turbines upwind
for i=1:length(p.farm.x)
    if(p.farm.x(i)<p.wt.x) %only compute deficit effect from upstream turbines

        %compute the grid number for low meandering edge
        mn=floor((w_c(i)-p.wt.exprad(i))/p.grid.size)+1;
        m_min=max(mn,1); 
        %compute the grid number for the high meandering edge
        mx=floor((w_c(i)+p.wt.exprad(i))/p.grid.size)+1;
        m_max=min(mx,floor(p.grid.ysize/p.grid.size)+1);

        %Merge wakes
        for j=m_min:m_max
            av(j)=av(j)*a(i);
        end
    end
end
    


%Rotor grid points - Todo move to farm setup
%compute the grid number for low rotor edge
r_min=floor((p.wt.y-p.wt.rotor.r)/p.grid.size)+1; 
%compute the grid number for the high rotor edge
r_max=floor((p.wt.y+p.wt.rotor.r)/p.grid.size)+1;

%Sum the wake corrected x-velocities over the rotor dish area
for i=r_min:r_max
    uxsum=uxsum+ux(i)*av(i);
end

%Compute the average
uavg=uxsum/(r_max-r_min+1);

%Extract the wake corrected velocity at the nacelle
upoint=ux(floor(p.wt.y/p.grid.size)+1)*av(floor(p.wt.y/p.grid.size)+1);
    
end

== matlab function Wind Field/Wake7/Wake deficit and centerline ==
  data: uy input double; CT input double; p parameter double; w_c output double; a output double
function [w_c,a]=wake(uy,CT,p)
    % Todo: Change length(uy)*grid_size to farm size?
    %       Change such that parm is passed directly

    w_c=zeros(length(p.farm.x),1);
    a=ones(length(p.farm.x),1);
    
    %Find wake center and deficit for all other turbines
    for i=1:length(p.farm.x)
        if(p.farm.x(i)-p.wt.x>0) %Is turbine P downwind?
            w_c(i)=max(min(wakecenter(uy,p.farm.x(i),p),p.grid.ysize),1);
            a(i)=deficit(CT,p.farm.x(i),p);
        else
            w_c(i)=p.wt.y;
            a(i)=1;
        end
    end
end


function wc=wakecenter(uy,px,p)
    
    %computer number of grid points to px
    it=ceil((px-p.wt.x)/p.grid.size);
    
    wc=p.wt.y; %wake center starts at turbine position
    
    for i=1:it %Compute the meandering for each grid point
        uysum=0;
        %compute the grid number for low meandering edge
        m_min=max(floor((wc-p.wt.meand.r(i))/p.grid.size)+1,1); 
        %compute the grid number for the high meandering edge
        m_max=min(ceil((wc+p.wt.meand.r(i))/p.grid.size)+1,length(uy)); 
        
        %average transversal velocity over the wake area
        for j=m_min:m_max
            uysum=uysum+uy(j);
        end
        uyavg=uysum/(m_max-m_min+1);
        
        % wake center filtered
        % Should be calculated from mean air speed vs uyavg and lowpass filtered
        % Redo filter computation
        % yeff=ts*uyavg=grid.size/uxmean*uyavg
        % wc=wc+alpha*uyavg
        
        %Uncomment to reproduce bug
        %wc=p.wt.y;
        
        %Uncomment to reproduce meandering limits
        %wc=min(max(wc,p.wt.y-150),p.wt.y+150);
        
        %Notice here ts such that wake moves with uyavg speed
        wc=wc+p.ts*uyavg;
    end
end

function a=deficit(CT,px,p)
    %wake deficit at px-wtx distance from turbine
    a=1-.5*CT/(1+(px-p.wt.x)/(4*p.wt.rotor.r));
end

== matlab function Wind Field/Wind Speed7/Wind speed ==
  data: ux input double; w_c input double; a input double; p parameter double; uavg output double; upoint output double
function [uavg,upoint]=windspeed(ux,w_c,a,p)
% ux    is the wind vector at the x position of the turbine
% w_c   is the vector of wake centers from the other turbins at the x position of the turbine
% a     is the vector of velocity deficits at this turbine from the other turbines
% P     is a structure containing parameters  
   
%Initialise variables
uxsum=0;
av=ones(p.grid.ysize,1);

%Merge the wake from all turbines upwind
for i=1:length(p.farm.x)
    if(p.farm.x(i)<p.wt.x) %only compute deficit effect from upstream turbines

        %compute the grid number for low meandering edge
        mn=floor((w_c(i)-p.wt.exprad(i))/p.grid.size)+1;
        m_min=max(mn,1); 
        %compute the grid number for the high meandering edge
        mx=floor((w_c(i)+p.wt.exprad(i))/p.grid.size)+1;
        m_max=min(mx,floor(p.grid.ysize/p.grid.size)+1);

        %Merge wakes
        for j=m_min:m_max
            av(j)=av(j)*a(i);
        end
    end
end
    


%Rotor grid points - Todo move to farm setup
%compute the grid number for low rotor edge
r_min=floor((p.wt.y-p.wt.rotor.r)/p.grid.size)+1; 
%compute the grid number for the high rotor edge
r_max=floor((p.wt.y+p.wt.rotor.r)/p.grid.size)+1;

%Sum the wake corrected x-velocities over the rotor dish area
for i=r_min:r_max
    uxsum=uxsum+ux(i)*av(i);
end

%Compute the average
uavg=uxsum/(r_max-r_min+1);

%Extract the wake corrected velocity at the nacelle
upoint=ux(floor(p.wt.y/p.grid.size)+1)*av(floor(p.wt.y/p.grid.size)+1);
    
end

== matlab function Wind Field/Wake8/Wake deficit and centerline ==
  data: uy input double; CT input double; p parameter double; w_c output double; a output double
function [w_c,a]=wake(uy,CT,p)
    % Todo: Change length(uy)*grid_size to farm size?
    %       Change such that parm is passed directly

    w_c=zeros(length(p.farm.x),1);
    a=ones(length(p.farm.x),1);
    
    %Find wake center and deficit for all other turbines
    for i=1:length(p.farm.x)
        if(p.farm.x(i)-p.wt.x>0) %Is turbine P downwind?
            w_c(i)=max(min(wakecenter(uy,p.farm.x(i),p),p.grid.ysize),1);
            a(i)=deficit(CT,p.farm.x(i),p);
        else
            w_c(i)=p.wt.y;
            a(i)=1;
        end
    end
end


function wc=wakecenter(uy,px,p)
    
    %computer number of grid points to px
    it=ceil((px-p.wt.x)/p.grid.size);
    
    wc=p.wt.y; %wake center starts at turbine position
    
    for i=1:it %Compute the meandering for each grid point
        uysum=0;
        %compute the grid number for low meandering edge
        m_min=max(floor((wc-p.wt.meand.r(i))/p.grid.size)+1,1); 
        %compute the grid number for the high meandering edge
        m_max=min(ceil((wc+p.wt.meand.r(i))/p.grid.size)+1,length(uy)); 
        
        %average transversal velocity over the wake area
        for j=m_min:m_max
            uysum=uysum+uy(j);
        end
        uyavg=uysum/(m_max-m_min+1);
        
        % wake center filtered
        % Should be calculated from mean air speed vs uyavg and lowpass filtered
        % Redo filter computation
        % yeff=ts*uyavg=grid.size/uxmean*uyavg
        % wc=wc+alpha*uyavg
        
        %Uncomment to reproduce bug
        %wc=p.wt.y;
        
        %Uncomment to reproduce meandering limits
        %wc=min(max(wc,p.wt.y-150),p.wt.y+150);
        
        %Notice here ts such that wake moves with uyavg speed
        wc=wc+p.ts*uyavg;
    end
end

function a=deficit(CT,px,p)
    %wake deficit at px-wtx distance from turbine
    a=1-.5*CT/(1+(px-p.wt.x)/(4*p.wt.rotor.r));
end

== matlab function Wind Field/Wind Speed8/Wind speed ==
  data: ux input double; w_c input double; a input double; p parameter double; uavg output double; upoint output double
function [uavg,upoint]=windspeed(ux,w_c,a,p)
% ux    is the wind vector at the x position of the turbine
% w_c   is the vector of wake centers from the other turbins at the x position of the turbine
% a     is the vector of velocity deficits at this turbine from the other turbines
% P     is a structure containing parameters  
   
%Initialise variables
uxsum=0;
av=ones(p.grid.ysize,1);

%Merge the wake from all turbines upwind
for i=1:length(p.farm.x)
    if(p.farm.x(i)<p.wt.x) %only compute deficit effect from upstream turbines

        %compute the grid number for low meandering edge
        mn=floor((w_c(i)-p.wt.exprad(i))/p.grid.size)+1;
        m_min=max(mn,1); 
        %compute the grid number for the high meandering edge
        mx=floor((w_c(i)+p.wt.exprad(i))/p.grid.size)+1;
        m_max=min(mx,floor(p.grid.ysize/p.grid.size)+1);

        %Merge wakes
        for j=m_min:m_max
            av(j)=av(j)*a(i);
        end
    end
end
    


%Rotor grid points - Todo move to farm setup
%compute the grid number for low rotor edge
r_min=floor((p.wt.y-p.wt.rotor.r)/p.grid.size)+1; 
%compute the grid number for the high rotor edge
r_max=floor((p.wt.y+p.wt.rotor.r)/p.grid.size)+1;

%Sum the wake corrected x-velocities over the rotor dish area
for i=r_min:r_max
    uxsum=uxsum+ux(i)*av(i);
end

%Compute the average
uavg=uxsum/(r_max-r_min+1);

%Extract the wake corrected velocity at the nacelle
upoint=ux(floor(p.wt.y/p.grid.size)+1)*av(floor(p.wt.y/p.grid.size)+1);
    
end
